simulink model "PrkgClimaEveMgr"  (R2022b) (continued)
== system PrkgClimaEveMgr == (continued)
  ClimaCfgForPostCdnAvl1_ClimaCfgForPostCdnAvl1/1 -> PrkgClimaEveMgr_Runnable_sys/15(ClimaCfgForPostCdnAvl1_ClimaCfgForPostCdnAvl1_read)
  ClimaCfgForPreClng1_ClimaCfgForPreClng1/1 -> PrkgClimaEveMgr_Runnable_sys/16(ClimaCfgForPreClng1_ClimaCfgForPreClng1_read)
  ClimaCfgForVehElec1_ClimaCfgForVehElec1/1 -> PrkgClimaEveMgr_Runnable_sys/17(ClimaCfgForVehElec1_ClimaCfgForVehElec1_read)
  ClimaCfgForVehHyb1_ClimaCfgForVehHyb1/1 -> PrkgClimaEveMgr_Runnable_sys/18(ClimaCfgForVehHyb1_ClimaCfgForVehHyb1_read)
  ClimaCfgForVrntOfVehSysElec1_ClimaCfgForVrntOfVehSysElec1/1 -> PrkgClimaEveMgr_Runnable_sys/19(ClimaCfgForVrntOfVehSysElec1_ClimaCfgForVrntOfVehSysElec1_read)
  ClimaOvrHeatProWarn_ClimaOvrHeatProWarn/1 -> PrkgClimaEveMgr_Runnable_sys/20(ClimaOvrHeatProWarn_ClimaOvrHeatProWarn_read)
  ClimaOvrHeatReqFromExrsc_ClimaOvrHeatReqFromExrsc/1 -> PrkgClimaEveMgr_Runnable_sys/21(ClimaOvrHeatReqFromExrsc_ClimaOvrHeatReqFromExrsc_read)
  ClimaStrtTiDly_ClimaStrtTiDly/1 -> PrkgClimaEveMgr_Runnable_sys/22(ClimaStrtTiDly_ClimaStrtTiDly_read)
  ClimaTmrSts_ClimaTmrSts/1 -> PrkgClimaEveMgr_Runnable_sys/23(ClimaTmrSts_ClimaTmrSts_read)
  CmptmtClsd_CmptmtClsd/1 -> PrkgClimaEveMgr_Runnable_sys/24(CmptmtClsd_CmptmtClsd_read)
  COHSts_COHSts/1 -> PrkgClimaEveMgr_Runnable_sys/25(COHSts_COHSts_read)
  CooltTForPrkgClima_CooltTForPrkgClima/1 -> PrkgClimaEveMgr_Runnable_sys/26(CooltTForPrkgClima_CooltTForPrkgClima_read)
  DCChrgnHndlSts_DCChrgnHndlSts/1 -> PrkgClimaEveMgr_Runnable_sys/27(DCChrgnHndlSts_DCChrgnHndlSts_read)
  DlyReqForTmrForPrkgClima_DlyReqForTmrForPrkgClima/1 -> PrkgClimaEveMgr_Runnable_sys/28(DlyReqForTmrForPrkgClima_DlyReqForTmrForPrkgClima_read)
  DshbTForPrkgClima_DshbTForPrkgClima/1 -> PrkgClimaEveMgr_Runnable_sys/29(DshbTForPrkgClima_DshbTForPrkgClima_read)
  EngT_Ngcc_EngT/1 -> PrkgClimaEveMgr_Runnable_sys/30(EngT_Ngcc_EngT_read)
  ErsCmdByKeyFromExsrc_ErsCmdByKeyFromExsrc/1 -> PrkgClimaEveMgr_Runnable_sys/31(ErsCmdByKeyFromExsrc_ErsCmdByKeyFromExsrc_read)
  ErsRunTimeFromExtSrc_ErsRunTimeFromExtSrc/1 -> PrkgClimaEveMgr_Runnable_sys/32(ErsRunTimeFromExtSrc_ErsRunTimeFromExtSrc_read)
  HeatrElecNotAvlForTmr_HeatrElecNotAvlForTmr/1 -> PrkgClimaEveMgr_Runnable_sys/33(HeatrElecNotAvlForTmr_HeatrElecNotAvlForTmr_read)
  HeatrTypSetgForPrkgClima_HeatrTypSetgForPrkgClima/1 -> PrkgClimaEveMgr_Runnable_sys/34(HeatrTypSetgForPrkgClima_HeatrTypSetgForPrkgClima_read)
  HeatUpRqrdForPrkgClima_HeatUpRqrdForPrkgClima/1 -> PrkgClimaEveMgr_Runnable_sys/35(HeatUpRqrdForPrkgClima_HeatUpRqrdForPrkgClima_read)
  HMIExtVentFanVolReqFromExtSrc_HMIExtVentFanVolReqFromExtSrc/1 -> PrkgClimaEveMgr_Runnable_sys/36(HMIExtVentFanVolReqFromExtSrc_HMIExtVentFanVolReqFromExtSrc_read)
  HMIExtVentonoffReqFromExtSrc_HMIExtVentonoffReqFromExtSrc/1 -> PrkgClimaEveMgr_Runnable_sys/37(HMIExtVentonoffReqFromExtSrc_HMIExtVentonoffReqFromExtSrc_read)
  HMIExtVentTSpReqFromExtSrc_HMIExtVentTSpReqFromExtSrc/1 -> PrkgClimaEveMgr_Runnable_sys/38(HMIExtVentTSpReqFromExtSrc_HMIExtVentTSpReqFromExtSrc_read)
  HMIExtVentTStsReqFromExtSrc_HMIExtVentTStsReqFromExtSrc/1 -> PrkgClimaEveMgr_Runnable_sys/39(HMIExtVentTStsReqFromExtSrc_HMIExtVentTStsReqFromExtSrc_read)
  HvBattSoc_HvBattSoc/1 -> PrkgClimaEveMgr_Runnable_sys/40(HvBattSoc_HvBattSoc_read)
  HvSysCnctWoU_HvSysCnctWoU/1 -> PrkgClimaEveMgr_Runnable_sys/41(HvSysCnctWoU_HvSysCnctWoU_read)
  HvSysWoU_HvSysWoU/1 -> PrkgClimaEveMgr_Runnable_sys/42(HvSysWoU_HvSysWoU_read)
  IntCo2ActvSts_IntCo2ActvSts/1 -> PrkgClimaEveMgr_Runnable_sys/43(IntCo2ActvSts_IntCo2ActvSts_read)
  IntCo2fromExsrc_IntCo2fromExsrc/1 -> PrkgClimaEveMgr_Runnable_sys/44(IntCo2fromExsrc_IntCo2fromExsrc_read)
  KeyClimaActv_KeyClimaActv/1 -> PrkgClimaEveMgr_Runnable_sys/45(KeyClimaActv_KeyClimaActv_read)
  LockgCenSts_Ngcc_LockgCenSts/1 -> PrkgClimaEveMgr_Runnable_sys/46(LockgCenSts_Ngcc_LockgCenSts_read)
  OnBdChrgrHndlSts_OnBdChrgrHndlSts/1 -> PrkgClimaEveMgr_Runnable_sys/47(OnBdChrgrHndlSts_OnBdChrgrHndlSts_read)
  PostDrvgClimaReq_Ngcc_PostDrvgClimaReq/1 -> PrkgClimaEveMgr_Runnable_sys/48(PostDrvgClimaReq_Ngcc_PostDrvgClimaReq_read)
  PreClngRqrdFromExtSrc_PreClngRqrdFromExtSrc/1 -> PrkgClimaEveMgr_Runnable_sys/49(PreClngRqrdFromExtSrc_PreClngRqrdFromExtSrc_read)
  PrkgClimaDiByCarMod_PrkgClimaDiByCarMod/1 -> PrkgClimaEveMgr_Runnable_sys/50(PrkgClimaDiByCarMod_PrkgClimaDiByCarMod_read)
  PrkgClimaRqrdImdtForBattULo_PrkgClimaRqrdImdtForBattULo/1 -> PrkgClimaEveMgr_Runnable_sys/51(PrkgClimaRqrdImdtForBattULo_PrkgClimaRqrdImdtForBattULo_read)
  PrkgClimaRqrdImdtFromActvMgr_PrkgClimaRqrdImdtFromActvMgr/1 -> PrkgClimaEveMgr_Runnable_sys/52(PrkgClimaRqrdImdtFromActvMgr_PrkgClimaRqrdImdtFromActvMgr_read)
  PrkgClimaRqrdImdtFromExtSrc_PrkgClimaRqrdImdtFromExtSrc/1 -> PrkgClimaEveMgr_Runnable_sys/53(PrkgClimaRqrdImdtFromExtSrc_PrkgClimaRqrdImdtFromExtSrc_read)
  PrkgClimaRqrdTmrForBattULo_PrkgClimaRqrdTmrForBattULo/1 -> PrkgClimaEveMgr_Runnable_sys/54(PrkgClimaRqrdTmrForBattULo_PrkgClimaRqrdTmrForBattULo_read)
  RemClimaFnshd_RemClimaFnshd/1 -> PrkgClimaEveMgr_Runnable_sys/55(RemClimaFnshd_RemClimaFnshd_read)
  RemClimaReqFromExtSrc_RemClimaReqFromExtSrc/1 -> PrkgClimaEveMgr_Runnable_sys/56(RemClimaReqFromExtSrc_RemClimaReqFromExtSrc_read)
  RemStrtExtnTiReqFromExtSrc_RemStrtExtnTiReqFromExtSrc/1 -> PrkgClimaEveMgr_Runnable_sys/57(RemStrtExtnTiReqFromExtSrc_RemStrtExtnTiReqFromExtSrc_read)
  RemStrtHvCtrlReqFromExtSrc_RemStrtHvCtrlReqFromExtSrc/1 -> PrkgClimaEveMgr_Runnable_sys/58(RemStrtHvCtrlReqFromExtSrc_RemStrtHvCtrlReqFromExtSrc_read)
  RemVentFbSts_RemVentFbSts/1 -> PrkgClimaEveMgr_Runnable_sys/59(RemVentFbSts_RemVentFbSts_read)
  RemVentReqfromExsrc_RemVentReqfromExsrc/1 -> PrkgClimaEveMgr_Runnable_sys/60(RemVentReqfromExsrc_RemVentReqfromExsrc_read)
  ReqForPrkgClima_ReqForPrkgClima/1 -> PrkgClimaEveMgr_Runnable_sys/61(ReqForPrkgClima_ReqForPrkgClima_read)
  ReqForSwtHeatrTypFromActvMgr_ReqForSwtHeatrTypFromActvMgr/1 -> PrkgClimaEveMgr_Runnable_sys/62(ReqForSwtHeatrTypFromActvMgr_ReqForSwtHeatrTypFromActvMgr_read)
  RtSdlForPrkgClima_RtSdlForPrkgClima/1 -> PrkgClimaEveMgr_Runnable_sys/63(RtSdlForPrkgClima_RtSdlForPrkgClima_read)
  SeatHeatgEnadFromHmi_SeatHeatgEnadFromHmi/1 -> PrkgClimaEveMgr_Runnable_sys/64(SeatHeatgEnadFromHmi_SeatHeatgEnadFromHmi_read)
  SeatHeatgEnadFromTelm_SeatHeatgEnadFromTelm/1 -> PrkgClimaEveMgr_Runnable_sys/65(SeatHeatgEnadFromTelm_SeatHeatgEnadFromTelm_read)
  SnsrQfOkForPrkgClima_SnsrQfOkForPrkgClima/1 -> PrkgClimaEveMgr_Runnable_sys/66(SnsrQfOkForPrkgClima_SnsrQfOkForPrkgClima_read)
  SteerWhlHeatgDurgClimaEnadFromExsrc_SteerWhlHeatgDurgClimaEnadFromExsrc/1 -> PrkgClimaEveMgr_Runnable_sys/67(SteerWhlHeatgDurgClimaEnadFromExsrc_SteerWhlHeatgDurgClimaEnadFromExsrc_read)
  SteerWhlHeatgEnadFromHmi_SteerWhlHeatgEnadFromHmi/1 -> PrkgClimaEveMgr_Runnable_sys/68(SteerWhlHeatgEnadFromHmi_SteerWhlHeatgEnadFromHmi_read)
  SteerWhlHeatgEnadFromTelm_SteerWhlHeatgEnadFromTelm/1 -> PrkgClimaEveMgr_Runnable_sys/69(SteerWhlHeatgEnadFromTelm_SteerWhlHeatgEnadFromTelm_read)
  StrtRemStsFromExsrc_StrtRemStsFromExsrc/1 -> PrkgClimaEveMgr_Runnable_sys/70(StrtRemStsFromExsrc_StrtRemStsFromExsrc_read)
  SuspStrtActvForPrkgClima_SuspStrtActvForPrkgClima/1 -> PrkgClimaEveMgr_Runnable_sys/71(SuspStrtActvForPrkgClima_SuspStrtActvForPrkgClima_read)
  SysDogSlpCountTi_SysDogSlpCountTi/1 -> PrkgClimaEveMgr_Runnable_sys/72(SysDogSlpCountTi_SysDogSlpCountTi_read)
  TelmClimaExtVentnLvlFromExtSrc_TelmClimaExtVentnLvlFromExtSrc/1 -> PrkgClimaEveMgr_Runnable_sys/73(TelmClimaExtVentnLvlFromExtSrc_TelmClimaExtVentnLvlFromExtSrc_read)
  TelmClimaExtVentnReqFromExtSrc_TelmClimaExtVentnReqFromExtSrc/1 -> PrkgClimaEveMgr_Runnable_sys/74(TelmClimaExtVentnReqFromExtSrc_TelmClimaExtVentnReqFromExtSrc_read)
  TelmClimaExtVentnTempRangeFromExtSrc_TelmClimaExtVentnTempRangeFromExtSrc/1 -> PrkgClimaEveMgr_Runnable_sys/75(TelmClimaExtVentnTempRangeFromExtSrc_TelmClimaExtVentnTempRangeFromExtSrc_read)
  TelmClimaExtVentnTSpSpclFromExtSrc_TelmClimaExtVentnTSpSpclFromExtSrc/1 -> PrkgClimaEveMgr_Runnable_sys/76(TelmClimaExtVentnTSpSpclFromExtSrc_TelmClimaExtVentnTSpSpclFromExtSrc_read)
  TelmClimaTmrFromExtSrc_TelmClimaTmrFromExtSrc/1 -> PrkgClimaEveMgr_Runnable_sys/77(TelmClimaTmrFromExtSrc_TelmClimaTmrFromExtSrc_read)
  TelmSeatDrvrHeatReqFromExsrc_TelmSeatDrvrHeatReqFromExsrc/1 -> PrkgClimaEveMgr_Runnable_sys/78(TelmSeatDrvrHeatReqFromExsrc_TelmSeatDrvrHeatReqFromExsrc_read)
  TelmSeatDrvVentnReqFromExsrc_TelmSeatDrvVentnReqFromExsrc/1 -> PrkgClimaEveMgr_Runnable_sys/79(TelmSeatDrvVentnReqFromExsrc_TelmSeatDrvVentnReqFromExsrc_read)
  TelmSeatPassHeatReqFromExsrc_TelmSeatPassHeatReqFromExsrc/1 -> PrkgClimaEveMgr_Runnable_sys/80(TelmSeatPassHeatReqFromExsrc_TelmSeatPassHeatReqFromExsrc_read)
  TelmSeatPassVentnReqFromExsrc_TelmSeatPassVentnReqFromExsrc/1 -> PrkgClimaEveMgr_Runnable_sys/81(TelmSeatPassVentnReqFromExsrc_TelmSeatPassVentnReqFromExsrc_read)
  TelmSeatSecLeHeatReqFromExsrc_TelmSeatSecLeHeatReqFromExsrc/1 -> PrkgClimaEveMgr_Runnable_sys/82(TelmSeatSecLeHeatReqFromExsrc_TelmSeatSecLeHeatReqFromExsrc_read)
  TelmSeatSecLeVentnReqFromExsrc_TelmSeatSecLeVentnReqFromExsrc/1 -> PrkgClimaEveMgr_Runnable_sys/83(TelmSeatSecLeVentnReqFromExsrc_TelmSeatSecLeVentnReqFromExsrc_read)
  TelmSeatSecRiHeatReqFromExsrc_TelmSeatSecRiHeatReqFromExsrc/1 -> PrkgClimaEveMgr_Runnable_sys/84(TelmSeatSecRiHeatReqFromExsrc_TelmSeatSecRiHeatReqFromExsrc_read)
  TelmSeatSecRiVentnReqFromExsrc_TelmSeatSecRiVentnReqFromExsrc/1 -> PrkgClimaEveMgr_Runnable_sys/85(TelmSeatSecRiVentnReqFromExsrc_TelmSeatSecRiVentnReqFromExsrc_read)
  TForHeatrPreCdngTyp_TForHeatrPreCdngTyp/1 -> PrkgClimaEveMgr_Runnable_sys/86(TForHeatrPreCdngTyp_TForHeatrPreCdngTyp_read)
  TmrForPrkgClima_TmrForPrkgClima/1 -> PrkgClimaEveMgr_Runnable_sys/87(TmrForPrkgClima_TmrForPrkgClima_read)
  VehModMngtGlbSafe1_Ngcc_VehModMngtGlbSafe1/1 -> PrkgClimaEveMgr_Runnable_sys/88(VehModMngtGlbSafe1_Ngcc_VehModMngtGlbSafe1_read)
  VehSunLoadTot_VehSunLoadTot/1 -> PrkgClimaEveMgr_Runnable_sys/89(VehSunLoadTot_VehSunLoadTot_read)
  VehSunLoadTotFild_VehSunLoadTotFild/1 -> PrkgClimaEveMgr_Runnable_sys/90(VehSunLoadTotFild_VehSunLoadTotFild_read)
  ClimaCfgForStatrtDevice_ClimaCfgForStartDevice/1 -> PrkgClimaEveMgr_Runnable_sys/91(ClimaCfgForStatrtDevice_ClimaCfgForStartDevice_read)
  PostHeatgRunTiEstimd_PostHeatgRunTiEstimd/1 -> PrkgClimaEveMgr_Runnable_sys/92(PostHeatgRunTiEstimd_PostHeatgRunTiEstimd_read)
  TelmDefrostReqFromExsrc_TelmDefrostReqFromExsrc/1 -> PrkgClimaEveMgr_Runnable_sys/93(TelmDefrostReqFromExsrc_TelmDefrostReqFromExsrc_read)
  TelmSeatThrdLeHeatReqFromExsrc_TelmSeatThrdLeHeatReqFromExsrc/1 -> PrkgClimaEveMgr_Runnable_sys/94(TelmSeatThrdLeHeatReqFromExsrc_TelmSeatThrdLeHeatReqFromExsrc_read)
  TelmSeatThrdLeVentnReqFromExsrc_TelmSeatThrdLeVentnReqFromExsrc/1 -> PrkgClimaEveMgr_Runnable_sys/95(TelmSeatThrdLeVentnReqFromExsrc_TelmSeatThrdLeVentnReqFromExsrc_read)
  TelmSeatThrdRiHeatReqFromExsrc_TelmSeatThrdRiHeatReqFromExsrc/1 -> PrkgClimaEveMgr_Runnable_sys/96(TelmSeatThrdRiHeatReqFromExsrc_TelmSeatThrdRiHeatReqFromExsrc_read)
  TelmSeatThrdRiVentnReqFromExsrc_TelmSeatThrdRiVentnReqFromExsrc/1 -> PrkgClimaEveMgr_Runnable_sys/97(TelmSeatThrdRiVentnReqFromExsrc_TelmSeatThrdRiVentnReqFromExsrc_read)
  TempRangeFromExtSrc_TempRangeFromExtSrc/1 -> PrkgClimaEveMgr_Runnable_sys/98(TempRangeFromExtSrc_TempRangeFromExtSrc_read)
  CmptmtTFrntRaw_CmptmtTFrntRaw/1 -> PrkgClimaEveMgr_Runnable_sys/99(CmptmtTFrntRaw_CmptmtTFrntRaw_read)
  BookTrvlClimaActvReq2FromExSrc_BookTrvlClimaActvReq2FromExSrc/1 -> PrkgClimaEveMgr_Runnable_sys/100(BookTrvlClimaActvReq2FromExSrc_BookTrvlClimaActvReq2FromExSrc_read)
  BookTrvlClimaActvReq3FromExSrc_BookTrvlClimaActvReq3FromExSrc/1 -> PrkgClimaEveMgr_Runnable_sys/101(BookTrvlClimaActvReq3FromExSrc_BookTrvlClimaActvReq3FromExSrc_read)
  BookTrvlClimaActvReq4FromExSrc_BookTrvlClimaActvReq4FromExSrc/1 -> PrkgClimaEveMgr_Runnable_sys/102(BookTrvlClimaActvReq4FromExSrc_BookTrvlClimaActvReq4FromExSrc_read)
  BookTrvlClimaActvReq5FromExSrc_BookTrvlClimaActvReq5FromExSrc/1 -> PrkgClimaEveMgr_Runnable_sys/103(BookTrvlClimaActvReq5FromExSrc_BookTrvlClimaActvReq5FromExSrc_read)
  BookTrvlClimaActvReq6FromExSrc_BookTrvlClimaActvReq6FromExSrc/1 -> PrkgClimaEveMgr_Runnable_sys/104(BookTrvlClimaActvReq6FromExSrc_BookTrvlClimaActvReq6FromExSrc_read)
  BookTrvlClimaActvReq7FromExSrc_BookTrvlClimaActvReq7FromExSrc/1 -> PrkgClimaEveMgr_Runnable_sys/105(BookTrvlClimaActvReq7FromExSrc_BookTrvlClimaActvReq7FromExSrc_read)
  BookTrvlClimaFnshd_BookTrvlClimaFnshd/1 -> PrkgClimaEveMgr_Runnable_sys/106(BookTrvlClimaFnshd_BookTrvlClimaFnshd_read)
  ClimaActv_Ngcc_ClimaActv/1 -> PrkgClimaEveMgr_Runnable_sys/107(ClimaActv_Ngcc_ClimaActv_read)
  ClimaCfgForAirMtrlSnsrPrsnt1_ClimaCfgForAirMtrlSnsrPrsnt1/1 -> PrkgClimaEveMgr_Runnable_sys/108(ClimaCfgForAirMtrlSnsrPrsnt1_ClimaCfgForAirMtrlSnsrPrsnt1_read)
  ClimaCfgForBRAND_ClimaCfgForBRAND/1 -> PrkgClimaEveMgr_Runnable_sys/109(ClimaCfgForBRAND_ClimaCfgForBRAND_read)
  ClimaCfgForCabinOxgenSupply_ClimaCfgForCabinOxgenSupply/1 -> PrkgClimaEveMgr_Runnable_sys/110(ClimaCfgForCabinOxgenSupply_ClimaCfgForCabinOxgenSupply_read)
  ClimaCfgForHvacTyp_ClimaCfgForHvacTyp/1 -> PrkgClimaEveMgr_Runnable_sys/111(ClimaCfgForHvacTyp_ClimaCfgForHvacTyp_read)
  ClimaCfgForLifeDetection_ClimaCfgForLifeDetection/1 -> PrkgClimaEveMgr_Runnable_sys/112(ClimaCfgForLifeDetection_ClimaCfgForLifeDetection_read)
  ClimaCfgForPrpsnTyp_ClimaCfgForPrpsnTyp/1 -> PrkgClimaEveMgr_Runnable_sys/113(ClimaCfgForPrpsnTyp_ClimaCfgForPrpsnTyp_read)
  ClimaCfgForRFKEY_ClimaCfgForRFKEY/1 -> PrkgClimaEveMgr_Runnable_sys/114(ClimaCfgForRFKEY_ClimaCfgForRFKEY_read)
  ClimaCfgForSnsrHumPrsnt1_ClimaCfgForSnsrHumPrsnt1/1 -> PrkgClimaEveMgr_Runnable_sys/115(ClimaCfgForSnsrHumPrsnt1_ClimaCfgForSnsrHumPrsnt1_read)
  ClimaCfgForVehTyp_ClimaCfgForVehTyp/1 -> PrkgClimaEveMgr_Runnable_sys/116(ClimaCfgForVehTyp_ClimaCfgForVehTyp_read)
  ClimaWakUpTiDif1FromExSrc_ClimaWakUpTiDif1FromExSrc/1 -> PrkgClimaEveMgr_Runnable_sys/117(ClimaWakUpTiDif1FromExSrc_ClimaWakUpTiDif1FromExSrc_read)
  ClimaWakUpTiDif2FromExSrc_ClimaWakUpTiDif2FromExSrc/1 -> PrkgClimaEveMgr_Runnable_sys/118(ClimaWakUpTiDif2FromExSrc_ClimaWakUpTiDif2FromExSrc_read)
  ClimaWakUpTiDif3FromExSrc_ClimaWakUpTiDif3FromExSrc/1 -> PrkgClimaEveMgr_Runnable_sys/119(ClimaWakUpTiDif3FromExSrc_ClimaWakUpTiDif3FromExSrc_read)
  ClimaWakUpTiDif4FromExSrc_ClimaWakUpTiDif4FromExSrc/1 -> PrkgClimaEveMgr_Runnable_sys/120(ClimaWakUpTiDif4FromExSrc_ClimaWakUpTiDif4FromExSrc_read)
  ClimaWakUpTiDif5FromExSrc_ClimaWakUpTiDif5FromExSrc/1 -> PrkgClimaEveMgr_Runnable_sys/121(ClimaWakUpTiDif5FromExSrc_ClimaWakUpTiDif5FromExSrc_read)
  ClimaWakUpTiDif6FromExSrc_ClimaWakUpTiDif6FromExSrc/1 -> PrkgClimaEveMgr_Runnable_sys/122(ClimaWakUpTiDif6FromExSrc_ClimaWakUpTiDif6FromExSrc_read)
  ClimaWakUpTiDif7FromExSrc_ClimaWakUpTiDif7FromExSrc/1 -> PrkgClimaEveMgr_Runnable_sys/123(ClimaWakUpTiDif7FromExSrc_ClimaWakUpTiDif7FromExSrc_read)
  CmptmtAirTEstimdAtRowFirst_CmptmtAirTEstimdAtRowFirst/1 -> PrkgClimaEveMgr_Runnable_sys/124(CmptmtAirTEstimdAtRowFirst_CmptmtAirTEstimdAtRowFirst_read)
  CmptmtClngActv_CmptmtClngActv/1 -> PrkgClimaEveMgr_Runnable_sys/125(CmptmtClngActv_CmptmtClngActv_read)
  CmptmtRelHum_Ngcc_CmptmtRelHum/1 -> PrkgClimaEveMgr_Runnable_sys/126(CmptmtRelHum_Ngcc_CmptmtRelHum_read)
  CmptmtTRearRaw_CmptmtTRearRaw/1 -> PrkgClimaEveMgr_Runnable_sys/127(CmptmtTRearRaw_CmptmtTRearRaw_read)
  DoorOpenStsForClima_DoorOpenStsForClima/1 -> PrkgClimaEveMgr_Runnable_sys/128(DoorOpenStsForClima_DoorOpenStsForClima_read)
  DoorStsWithOutTrForClima_DoorStsWithOutTrForClima/1 -> PrkgClimaEveMgr_Runnable_sys/129(DoorStsWithOutTrForClima_DoorStsWithOutTrForClima_read)
  EngSt1WdStsFromExsrc_EngSt1WdStsFromExsrc/1 -> PrkgClimaEveMgr_Runnable_sys/130(EngSt1WdStsFromExsrc_EngSt1WdStsFromExsrc_read)
  FrntHvacBlowerSts_FrntHvacBlowerSts/1 -> PrkgClimaEveMgr_Runnable_sys/131(FrntHvacBlowerSts_FrntHvacBlowerSts_read)
  GearLvrIndcn_GearLvrIndcn/1 -> PrkgClimaEveMgr_Runnable_sys/132(GearLvrIndcn_GearLvrIndcn_read)
  GearLvrIndcn_GearLvrIndcn_IsUpdated/1 -> PrkgClimaEveMgr_Runnable_sys/133(GearLvrIndcn_GearLvrIndcn_IsUpdated_read)
  HmiWinterLockCarPrevnIceSetNgcc_HmiWinterLockCarPrevnIceSetNgcc/1 -> PrkgClimaEveMgr_Runnable_sys/134(HmiWinterLockCarPrevnIceSetNgcc_HmiWinterLockCarPrevnIceSetNgcc_read)
  HvBattMntnReq_HvBattMntnReq/1 -> PrkgClimaEveMgr_Runnable_sys/135(HvBattMntnReq_HvBattMntnReq_read)
  HvBattMntnReq_HvBattMntnReq_IsUpdated/1 -> PrkgClimaEveMgr_Runnable_sys/136(HvBattMntnReq_HvBattMntnReq_IsUpdated_read)
  InflateAirSrcSwtNgcc_InflateAirSrcSwtNgcc/1 -> PrkgClimaEveMgr_Runnable_sys/137(InflateAirSrcSwtNgcc_InflateAirSrcSwtNgcc_read)
  KeyClimaActvFnshd_KeyClimaActvFnshd/1 -> PrkgClimaEveMgr_Runnable_sys/138(KeyClimaActvFnshd_KeyClimaActvFnshd_read)
  LonglifeBatteryReqNgcc_LonglifeBatteryReqNgcc/1 -> PrkgClimaEveMgr_Runnable_sys/139(LonglifeBatteryReqNgcc_LonglifeBatteryReqNgcc_read)
  MemReadForPrkgClimaEveMgr_MemReadForPrkgClimaEveMgr/1 -> PrkgClimaEveMgr_Runnable_sys/140(MemReadForPrkgClimaEveMgr_MemReadForPrkgClimaEveMgr_read)
  OGMAirSrcFnshd_OGMAirSrcFnshd/1 -> PrkgClimaEveMgr_Runnable_sys/141(OGMAirSrcFnshd_OGMAirSrcFnshd_read)
  PrvntWinFrz_PrvntWinFrz/1 -> PrkgClimaEveMgr_Runnable_sys/142(PrvntWinFrz_PrvntWinFrz_read)
  PrvntWinFrzFnshd_PrvntWinFrzFnshd/1 -> PrkgClimaEveMgr_Runnable_sys/143(PrvntWinFrzFnshd_PrvntWinFrzFnshd_read)
  RemClimaFnshd1_RemClimaFnshd1/1 -> PrkgClimaEveMgr_Runnable_sys/144(RemClimaFnshd1_RemClimaFnshd1_read)
  RemClimaWarn_RemClimaWarn/1 -> PrkgClimaEveMgr_Runnable_sys/145(RemClimaWarn_RemClimaWarn_read)
  RemOxygenSplyFnshd_RemOxygenSplyFnshd/1 -> PrkgClimaEveMgr_Runnable_sys/146(RemOxygenSplyFnshd_RemOxygenSplyFnshd_read)
  RemOxygenSplyFnshd1_RemOxygenSplyFnshd1/1 -> PrkgClimaEveMgr_Runnable_sys/147(RemOxygenSplyFnshd1_RemOxygenSplyFnshd1_read)
  RemOxygenSplyWarn_RemOxygenSplyWarn/1 -> PrkgClimaEveMgr_Runnable_sys/148(RemOxygenSplyWarn_RemOxygenSplyWarn_read)
  TcsOvrheatdProtnStsNgcc_TcsOvrheatdProtnStsNgcc/1 -> PrkgClimaEveMgr_Runnable_sys/149(TcsOvrheatdProtnStsNgcc_TcsOvrheatdProtnStsNgcc_read)
  TelmBookClimaReqHmiCmptmtTSpSpclFromExtSrc_TelmBookClimaReqHmiCmptmtTSpSpclFromExtSrc/1 -> PrkgClimaEveMgr_Runnable_sys/150(TelmBookClimaReqHmiCmptmtTSpSpclFromExtSrc_TelmBookClimaReqHmiCmptmtTSpSpclFromExtSrc_read)
  TelmBookClimaReqOnOffNoReqFromExtSrc_TelmBookClimaReqOnOffNoReqFromExtSrc/1 -> PrkgClimaEveMgr_Runnable_sys/151(TelmBookClimaReqOnOffNoReqFromExtSrc_TelmBookClimaReqOnOffNoReqFromExtSrc_read)
  TelmBookClimaReqTempRangeFromExtSrc_TelmBookClimaReqTempRangeFromExtSrc/1 -> PrkgClimaEveMgr_Runnable_sys/152(TelmBookClimaReqTempRangeFromExtSrc_TelmBookClimaReqTempRangeFromExtSrc_read)
  TelmBookClimaTmrFromExtSrc_TelmBookClimaTmrFromExtSrc/1 -> PrkgClimaEveMgr_Runnable_sys/153(TelmBookClimaTmrFromExtSrc_TelmBookClimaTmrFromExtSrc_read)
  TelmBookSeatHeatClimalReqDrvHeatClimaLvlFromExtSrc_TelmBookSeatHeatClimalReqDrvHeatClimaLvlFromExtSrc/1 -> PrkgClimaEveMgr_Runnable_sys/154(TelmBookSeatHeatClimalReqDrvHeatClimaLvlFromExtSrc_TelmBookSeatHeatClimalReqDrvHeatClimaLvlFromExtSrc_read)
  TelmBookSeatHeatClimalReqPassHeatClimaLvlFromExtSrc_TelmBookSeatHeatClimalReqPassHeatClimaLvlFromExtSrc/1 -> PrkgClimaEveMgr_Runnable_sys/155(TelmBookSeatHeatClimalReqPassHeatClimaLvlFromExtSrc_TelmBookSeatHeatClimalReqPassHeatClimaLvlFromExtSrc_read)
  TelmBookSeatHeatClimalReqSecLeHeatClimaLvlFromExtSrc_TelmBookSeatHeatClimalReqSecLeHeatClimaLvlFromExtSrc/1 -> PrkgClimaEveMgr_Runnable_sys/156(TelmBookSeatHeatClimalReqSecLeHeatClimaLvlFromExtSrc_TelmBookSeatHeatClimalReqSecLeHeatClimaLvlFromExtSrc_read)
  TelmBookSeatHeatClimalReqSecRiHeatClimaLvlFromExtSrc_TelmBookSeatHeatClimalReqSecRiHeatClimaLvlFromExtSrc/1 -> PrkgClimaEveMgr_Runnable_sys/157(TelmBookSeatHeatClimalReqSecRiHeatClimaLvlFromExtSrc_TelmBookSeatHeatClimalReqSecRiHeatClimaLvlFromExtSrc_read)
  TelmBookSeatHeatClimalReqThrdLeHeatClimaLvlFromExtSrc_TelmBookSeatHeatClimalReqThrdLeHeatClimaLvlFromExtSrc/1 -> PrkgClimaEveMgr_Runnable_sys/158(TelmBookSeatHeatClimalReqThrdLeHeatClimaLvlFromExtSrc_TelmBookSeatHeatClimalReqThrdLeHeatClimaLvlFromExtSrc_read)
  TelmBookSeatHeatClimalReqThrdRiHeatClimaLvlFromExtSrc_TelmBookSeatHeatClimalReqThrdRiHeatClimaLvlFromExtSrc/1 -> PrkgClimaEveMgr_Runnable_sys/159(TelmBookSeatHeatClimalReqThrdRiHeatClimaLvlFromExtSrc_TelmBookSeatHeatClimalReqThrdRiHeatClimaLvlFromExtSrc_read)
  TelmBookSeatVentnClimalReqDrvVentnClimaLvlFromExtSrc_TelmBookSeatVentnClimalReqDrvVentnClimaLvlFromExtSrc/1 -> PrkgClimaEveMgr_Runnable_sys/160(TelmBookSeatVentnClimalReqDrvVentnClimaLvlFromExtSrc_TelmBookSeatVentnClimalReqDrvVentnClimaLvlFromExtSrc_read)
  TelmBookSeatVentnClimalReqPassVentnClimaLvlFromExtSrc_TelmBookSeatVentnClimalReqPassVentnClimaLvlFromExtSrc/1 -> PrkgClimaEveMgr_Runnable_sys/161(TelmBookSeatVentnClimalReqPassVentnClimaLvlFromExtSrc_TelmBookSeatVentnClimalReqPassVentnClimaLvlFromExtSrc_read)
  TelmBookSeatVentnClimalReqSecLeVentnClimaLvlFromExtSrc_TelmBookSeatVentnClimalReqSecLeVentnClimaLvlFromExtSrc/1 -> PrkgClimaEveMgr_Runnable_sys/162(TelmBookSeatVentnClimalReqSecLeVentnClimaLvlFromExtSrc_TelmBookSeatVentnClimalReqSecLeVentnClimaLvlFromExtSrc_read)
  TelmBookSeatVentnClimalReqSecRiVentnClimaLvlFromExtSrc_TelmBookSeatVentnClimalReqSecRiVentnClimaLvlFromExtSrc/1 -> PrkgClimaEveMgr_Runnable_sys/163(TelmBookSeatVentnClimalReqSecRiVentnClimaLvlFromExtSrc_TelmBookSeatVentnClimalReqSecRiVentnClimaLvlFromExtSrc_read)
  TelmBookSeatVentnClimalReqThrdLeVentnClimaLvlFromExtSrc_TelmBookSeatVentnClimalReqThrdLeVentnClimaLvlFromExtSrc/1 -> PrkgClimaEveMgr_Runnable_sys/164(TelmBookSeatVentnClimalReqThrdLeVentnClimaLvlFromExtSrc_TelmBookSeatVentnClimalReqThrdLeVentnClimaLvlFromExtSrc_read)
  TelmBookSeatVentnClimalReqThrdRiVentnClimaLvlFromExtSrc_TelmBookSeatVentnClimalReqThrdRiVentnClimaLvlFromExtSrc/1 -> PrkgClimaEveMgr_Runnable_sys/165(TelmBookSeatVentnClimalReqThrdRiVentnClimaLvlFromExtSrc_TelmBookSeatVentnClimalReqThrdRiVentnClimaLvlFromExtSrc_read)
  TelmBookSteerWhlHeatClimalReqFromExtSrc_TelmBookSteerWhlHeatClimalReqFromExtSrc/1 -> PrkgClimaEveMgr_Runnable_sys/166(TelmBookSteerWhlHeatClimalReqFromExtSrc_TelmBookSteerWhlHeatClimalReqFromExtSrc_read)
  TelmDefrostFnshd_TelmDefrostFnshd/1 -> PrkgClimaEveMgr_Runnable_sys/167(TelmDefrostFnshd_TelmDefrostFnshd_read)
  TelmOxygenReqFromExSrc_TelmOxygenReqFromExSrc/1 -> PrkgClimaEveMgr_Runnable_sys/168(TelmOxygenReqFromExSrc_TelmOxygenReqFromExSrc_read)
  TelmSeatHeatDrvrFnshd_TelmSeatHeatDrvrFnshd/1 -> PrkgClimaEveMgr_Runnable_sys/169(TelmSeatHeatDrvrFnshd_TelmSeatHeatDrvrFnshd_read)
  TelmSeatHeatPassFnshd_TelmSeatHeatPassFnshd/1 -> PrkgClimaEveMgr_Runnable_sys/170(TelmSeatHeatPassFnshd_TelmSeatHeatPassFnshd_read)
  TelmSeatHeatSecLeFnshd_TelmSeatHeatSecLeFnshd/1 -> PrkgClimaEveMgr_Runnable_sys/171(TelmSeatHeatSecLeFnshd_TelmSeatHeatSecLeFnshd_read)
  TelmSeatHeatSecRiFnshd_TelmSeatHeatSecRiFnshd/1 -> PrkgClimaEveMgr_Runnable_sys/172(TelmSeatHeatSecRiFnshd_TelmSeatHeatSecRiFnshd_read)
  TelmSeatHeatThrdLeFnshd_TelmSeatHeatThrdLeFnshd/1 -> PrkgClimaEveMgr_Runnable_sys/173(TelmSeatHeatThrdLeFnshd_TelmSeatHeatThrdLeFnshd_read)
  TelmSeatHeatThrdRiFnshd_TelmSeatHeatThrdRiFnshd/1 -> PrkgClimaEveMgr_Runnable_sys/174(TelmSeatHeatThrdRiFnshd_TelmSeatHeatThrdRiFnshd_read)
  TelmSeatVentnDrvrFnshd_TelmSeatVentnDrvrFnshd/1 -> PrkgClimaEveMgr_Runnable_sys/175(TelmSeatVentnDrvrFnshd_TelmSeatVentnDrvrFnshd_read)
  TelmSeatVentnPassFnshd_TelmSeatVentnPassFnshd/1 -> PrkgClimaEveMgr_Runnable_sys/176(TelmSeatVentnPassFnshd_TelmSeatVentnPassFnshd_read)
  TelmSeatVentnSecLeFnshd_TelmSeatVentnSecLeFnshd/1 -> PrkgClimaEveMgr_Runnable_sys/177(TelmSeatVentnSecLeFnshd_TelmSeatVentnSecLeFnshd_read)
  TelmSeatVentnSecRiFnshd_TelmSeatVentnSecRiFnshd/1 -> PrkgClimaEveMgr_Runnable_sys/178(TelmSeatVentnSecRiFnshd_TelmSeatVentnSecRiFnshd_read)
  TelmSeatVentnThrdLeFnshd_TelmSeatVentnThrdLeFnshd/1 -> PrkgClimaEveMgr_Runnable_sys/179(TelmSeatVentnThrdLeFnshd_TelmSeatVentnThrdLeFnshd_read)
  TelmSeatVentnThrdRiFnshd_TelmSeatVentnThrdRiFnshd/1 -> PrkgClimaEveMgr_Runnable_sys/180(TelmSeatVentnThrdRiFnshd_TelmSeatVentnThrdRiFnshd_read)
  TelmSteerWhlHeatFnshd_TelmSteerWhlHeatFnshd/1 -> PrkgClimaEveMgr_Runnable_sys/181(TelmSteerWhlHeatFnshd_TelmSteerWhlHeatFnshd_read)
  UnLckClimaActvReq_UnLckClimaActvReq/1 -> PrkgClimaEveMgr_Runnable_sys/182(UnLckClimaActvReq_UnLckClimaActvReq_read)
  UnLckHeatActvReq_UnLckHeatActvReq/1 -> PrkgClimaEveMgr_Runnable_sys/183(UnLckHeatActvReq_UnLckHeatActvReq_read)
  VentnForWinOpenGlbActv_VentnForWinOpenGlbActv/1 -> PrkgClimaEveMgr_Runnable_sys/184(VentnForWinOpenGlbActv_VentnForWinOpenGlbActv_read)
  VTMOverTProtectionFnshd_VTMOverTProtectionFnshd/1 -> PrkgClimaEveMgr_Runnable_sys/185(VTMOverTProtectionFnshd_VTMOverTProtectionFnshd_read)
  WarnLvlMsgNgcc_WarnLvlMsgNgcc/1 -> PrkgClimaEveMgr_Runnable_sys/186(WarnLvlMsgNgcc_WarnLvlMsgNgcc_read)
  WarnLvlMsgNgcc_WarnLvlMsgNgcc_IsUpdated/1 -> PrkgClimaEveMgr_Runnable_sys/187(WarnLvlMsgNgcc_WarnLvlMsgNgcc_IsUpdated_read)
  WarnLvlMsgUWB_WarnLvlMsgUWB/1 -> PrkgClimaEveMgr_Runnable_sys/188(WarnLvlMsgUWB_WarnLvlMsgUWB_read)
  WarnLvlMsgUWB_WarnLvlMsgUWB_IsUpdated/1 -> PrkgClimaEveMgr_Runnable_sys/189(WarnLvlMsgUWB_WarnLvlMsgUWB_IsUpdated_read)
  WinOpenLDACReq_WinOpenLDACReq/1 -> PrkgClimaEveMgr_Runnable_sys/190(WinOpenLDACReq_WinOpenLDACReq_read)
  WinOpenLDACReqUWB_WinOpenLDACReqUWB/1 -> PrkgClimaEveMgr_Runnable_sys/191(WinOpenLDACReqUWB_WinOpenLDACReqUWB_read)
  WinPosnStsAtDrvrReNgcc_WinPosnStsAtDrvrReNgcc/1 -> PrkgClimaEveMgr_Runnable_sys/192(WinPosnStsAtDrvrReNgcc_WinPosnStsAtDrvrReNgcc_read)
  WinPosnStsAtPassReNgcc_WinPosnStsAtPassRe/1 -> PrkgClimaEveMgr_Runnable_sys/193(WinPosnStsAtPassReNgcc_WinPosnStsAtPassRe_read)
  WinPosnStsDrv_WinPosnStsAtDrv/1 -> PrkgClimaEveMgr_Runnable_sys/194(WinPosnStsDrv_WinPosnStsAtDrv_read)
  WinPosnStsPass_WinPosnStsAtPass/1 -> PrkgClimaEveMgr_Runnable_sys/195(WinPosnStsPass_WinPosnStsAtPass_read)
  PrkgClimaEveMgr_Runnable_sys/1(HvBattMntnActv_HvBattMntnActv_write) -> HvBattMntnActv_HvBattMntnActv/1
  PrkgClimaEveMgr_Runnable_sys/2(LDACState_LDACState_write) -> LDACState_LDACState/1
  PrkgClimaEveMgr_Runnable_sys/3(MemBookTrvlClimaTi_MemBookTrvlClimaTi_write) -> MemBookTrvlClimaTi_MemBookTrvlClimaTi/1
  PrkgClimaEveMgr_Runnable_sys/4(MemSaveFlgForPrkgClimaEveMgr_MemSaveFlgForPrkgClimaEveMgr_write) -> MemSaveFlgForPrkgClimaEveMgr_MemSaveFlgForPrkgClimaEveMgr/1
  PrkgClimaEveMgr_Runnable_sys/5(MemWrForPrkgClimaEveMgr_MemWrForPrkgClimaEveMgr_write) -> MemWrForPrkgClimaEveMgr_MemWrForPrkgClimaEveMgr/1
  PrkgClimaEveMgr_Runnable_sys/6(PrkgClimaReqExcpSeat_PrkgClimaReqExcpSeat_write) -> PrkgClimaReqExcpSeat_PrkgClimaReqExcpSeat/1
  PrkgClimaEveMgr_Runnable_sys/7(PrkgClimaReqPrio_PrkgClimaReqPrio_write) -> PrkgClimaReqPrio_PrkgClimaReqPrio/1
  PrkgClimaEveMgr_Runnable_sys/8(PrkgOGMAirSrcReq_PrkgOGMAirSrcReq_write) -> PrkgOGMAirSrcReq_PrkgOGMAirSrcReq/1
  PrkgClimaEveMgr_Runnable_sys/9(PrvntWinActvReq_PrvntWinActvReq_write) -> PrvntWinActvReq_PrvntWinActvReq/1
  PrkgClimaEveMgr_Runnable_sys/10(RemClimaEgyExsrcReqPlus_RemClimaEgyExsrcReqPlus_write) -> RemClimaEgyExsrcReqPlus_RemClimaEgyExsrcReqPlus/1
  PrkgClimaEveMgr_Runnable_sys/11(RemOxygenSplyReq_RemOxygenSplyReq_write) -> RemOxygenSplyReq_RemOxygenSplyReq/1
  PrkgClimaEveMgr_Runnable_sys/12(TcamClcltTiFb1_TcamClcltTiFb1_write) -> TcamClcltTiFb1_TcamClcltTiFb1/1
  PrkgClimaEveMgr_Runnable_sys/13(TcamClcltTiFb2_TcamClcltTiFb2_write) -> TcamClcltTiFb2_TcamClcltTiFb2/1
  PrkgClimaEveMgr_Runnable_sys/14(TcamClcltTiFb3_TcamClcltTiFb3_write) -> TcamClcltTiFb3_TcamClcltTiFb3/1
  PrkgClimaEveMgr_Runnable_sys/15(TcamClcltTiFb4_TcamClcltTiFb4_write) -> TcamClcltTiFb4_TcamClcltTiFb4/1
  PrkgClimaEveMgr_Runnable_sys/16(TcamClcltTiFb5_TcamClcltTiFb5_write) -> TcamClcltTiFb5_TcamClcltTiFb5/1
  PrkgClimaEveMgr_Runnable_sys/17(TcamClcltTiFb6_TcamClcltTiFb6_write) -> TcamClcltTiFb6_TcamClcltTiFb6/1
  PrkgClimaEveMgr_Runnable_sys/18(TcamClcltTiFb7_TcamClcltTiFb7_write) -> TcamClcltTiFb7_TcamClcltTiFb7/1
  PrkgClimaEveMgr_Runnable_sys/19(TcsOvrheatdProtnReq_TcsOvrheatdProtnReq_write) -> TcsOvrheatdProtnReq_TcsOvrheatdProtnReq/1
  PrkgClimaEveMgr_Runnable_sys/20(TelmBookClimaReqHmiCmptmtTSpSpclStrtReq_TelmBookClimaReqHmiCmptmtTSpSpclStrtReq_write) -> TelmBookClimaReqHmiCmptmtTSpSpclStrtReq_TelmBookClimaReqHmiCmptmtTSpSpclStrtReq/1
  PrkgClimaEveMgr_Runnable_sys/21(TelmBookClimaReqOnOffNoReqStrtReq_TelmBookClimaReqOnOffNoReqStrtReq_write) -> TelmBookClimaReqOnOffNoReqStrtReq_TelmBookClimaReqOnOffNoReqStrtReq/1
  PrkgClimaEveMgr_Runnable_sys/22(TelmBookClimaReqTempRangeStrtReq_TelmBookClimaReqTempRangeStrtReq_write) -> TelmBookClimaReqTempRangeStrtReq_TelmBookClimaReqTempRangeStrtReq/1
  PrkgClimaEveMgr_Runnable_sys/23(TelmBookClimaTmrStrtReq_TelmBookClimaTmrStrtReq_write) -> TelmBookClimaTmrStrtReq_TelmBookClimaTmrStrtReq/1
  PrkgClimaEveMgr_Runnable_sys/24(TelmBookSeatHeatClimalReqDrvHeatClimaLvlStrtReq_TelmBookSeatHeatClimalReqDrvHeatClimaLvlStrtReq_write) -> TelmBookSeatHeatClimalReqDrvHeatClimaLvlStrtReq_TelmBookSeatHeatClimalReqDrvHeatClimaLvlStrtReq/1
  PrkgClimaEveMgr_Runnable_sys/25(TelmBookSeatHeatClimalReqPassHeatClimaLvlStrtReq_TelmBookSeatHeatClimalReqPassHeatClimaLvlStrtReq_write) -> TelmBookSeatHeatClimalReqPassHeatClimaLvlStrtReq_TelmBookSeatHeatClimalReqPassHeatClimaLvlStrtReq/1
  PrkgClimaEveMgr_Runnable_sys/26(TelmBookSeatHeatClimalReqSecLeHeatClimaLvlStrtReq_TelmBookSeatHeatClimalReqSecLeHeatClimaLvlStrtReq_write) -> TelmBookSeatHeatClimalReqSecLeHeatClimaLvlStrtReq_TelmBookSeatHeatClimalReqSecLeHeatClimaLvlStrtReq/1
  PrkgClimaEveMgr_Runnable_sys/27(TelmBookSeatHeatClimalReqSecRiHeatClimaLvlStrtReq_TelmBookSeatHeatClimalReqSecRiHeatClimaLvlStrtReq_write) -> TelmBookSeatHeatClimalReqSecRiHeatClimaLvlStrtReq_TelmBookSeatHeatClimalReqSecRiHeatClimaLvlStrtReq/1
  PrkgClimaEveMgr_Runnable_sys/28(TelmBookSeatHeatClimalReqThrdLeHeatClimaLvlStrtReq_TelmBookSeatHeatClimalReqThrdLeHeatClimaLvlStrtReq_write) -> TelmBookSeatHeatClimalReqThrdLeHeatClimaLvlStrtReq_TelmBookSeatHeatClimalReqThrdLeHeatClimaLvlStrtReq/1
  PrkgClimaEveMgr_Runnable_sys/29(TelmBookSeatHeatClimalReqThrdRiHeatClimaLvlStrtReq_TelmBookSeatHeatClimalReqThrdRiHeatClimaLvlStrtReq_write) -> TelmBookSeatHeatClimalReqThrdRiHeatClimaLvlStrtReq_TelmBookSeatHeatClimalReqThrdRiHeatClimaLvlStrtReq/1
  PrkgClimaEveMgr_Runnable_sys/30(TelmBookSeatVentnClimalReqDrvVentnClimaLvlStrtReq_TelmBookSeatVentnClimalReqDrvVentnClimaLvlStrtReq_write) -> TelmBookSeatVentnClimalReqDrvVentnClimaLvlStrtReq_TelmBookSeatVentnClimalReqDrvVentnClimaLvlStrtReq/1
  PrkgClimaEveMgr_Runnable_sys/31(TelmBookSeatVentnClimalReqPassVentnClimaLvlStrtReq_TelmBookSeatVentnClimalReqPassVentnClimaLvlStrtReq_write) -> TelmBookSeatVentnClimalReqPassVentnClimaLvlStrtReq_TelmBookSeatVentnClimalReqPassVentnClimaLvlStrtReq/1
  PrkgClimaEveMgr_Runnable_sys/32(TelmBookSeatVentnClimalReqSecLeVentnClimaLvlStrtReq_TelmBookSeatVentnClimalReqSecLeVentnClimaLvlStrtReq_write) -> TelmBookSeatVentnClimalReqSecLeVentnClimaLvlStrtReq_TelmBookSeatVentnClimalReqSecLeVentnClimaLvlStrtReq/1
  PrkgClimaEveMgr_Runnable_sys/33(TelmBookSeatVentnClimalReqSecRiVentnClimaLvlStrtReq_TelmBookSeatVentnClimalReqSecRiVentnClimaLvlStrtReq_write) -> TelmBookSeatVentnClimalReqSecRiVentnClimaLvlStrtReq_TelmBookSeatVentnClimalReqSecRiVentnClimaLvlStrtReq/1
  PrkgClimaEveMgr_Runnable_sys/34(TelmBookSeatVentnClimalReqThrdLeVentnClimaLvlStrtReq_TelmBookSeatVentnClimalReqThrdLeVentnClimaLvlStrtReq_write) -> TelmBookSeatVentnClimalReqThrdLeVentnClimaLvlStrtReq_TelmBookSeatVentnClimalReqThrdLeVentnClimaLvlStrtReq/1
  PrkgClimaEveMgr_Runnable_sys/35(TelmBookSeatVentnClimalReqThrdRiVentnClimaLvlStrtReq_TelmBookSeatVentnClimalReqThrdRiVentnClimaLvlStrtReq_write) -> TelmBookSeatVentnClimalReqThrdRiVentnClimaLvlStrtReq_TelmBookSeatVentnClimalReqThrdRiVentnClimaLvlStrtReq/1
  PrkgClimaEveMgr_Runnable_sys/36(TelmBookSteerWhlHeatClimalReqStrtReq_TelmBookSteerWhlHeatClimalReqStrtReq_write) -> TelmBookSteerWhlHeatClimalReqStrtReq_TelmBookSteerWhlHeatClimalReqStrtReq/1
  PrkgClimaEveMgr_Runnable_sys/37(TelmSeatVentnThrdReq_TelmSeatVentnThrdReq_write) -> TelmSeatVentnThrdReq_TelmSeatVentnThrdReq/1
  PrkgClimaEveMgr_Runnable_sys/38(UnLckClimaActvReqSt_UnLckClimaActvReqSt_write) -> UnLckClimaActvReqSt_UnLckClimaActvReqSt/1
  PrkgClimaEveMgr_Runnable_sys/39(UnLckHeatActvReqSt_UnLckHeatActvReqSt_write) -> UnLckHeatActvReqSt_UnLckHeatActvReqSt/1
  PrkgClimaEveMgr_Runnable_sys/40(ActvRqrdForPostPrkgClima_ActvRqrdForPostPrkgClima_write) -> ActvRqrdForPostPrkgClima_ActvRqrdForPostPrkgClima/1
  PrkgClimaEveMgr_Runnable_sys/41(ClimaOvrHeatProWarnIni_ClimaOvrHeatProWarnIni_write) -> ClimaOvrHeatProWarnIni_ClimaOvrHeatProWarnIni/1
  PrkgClimaEveMgr_Runnable_sys/42(ClimaOvrHeatStrtReq_ClimaOvrHeatStrtReq_write) -> ClimaOvrHeatStrtReq_ClimaOvrHeatStrtReq/1
  PrkgClimaEveMgr_Runnable_sys/43(ClimaStrtTi_ClimaStrtTi_write) -> ClimaStrtTi_ClimaStrtTi/1
  PrkgClimaEveMgr_Runnable_sys/44(CSDUsgChgeOffReq_CSDUsgChgeOffReq_write) -> CSDUsgChgeOffReq_CSDUsgChgeOffReq/1
  PrkgClimaEveMgr_Runnable_sys/45(CSDUsgChgeOnReq_CSDUsgChgeOnReq_write) -> CSDUsgChgeOnReq_CSDUsgChgeOnReq/1
  PrkgClimaEveMgr_Runnable_sys/46(EcuDogKeepActv_EcuDogKeepActv_write) -> EcuDogKeepActv_EcuDogKeepActv/1
  PrkgClimaEveMgr_Runnable_sys/47(EcuDogSlpReq_EcuDogSlpReq_write) -> EcuDogSlpReq_EcuDogSlpReq/1
  PrkgClimaEveMgr_Runnable_sys/48(EcuDogSlpSetTi_EcuDogSlpSetTi_write) -> EcuDogSlpSetTi_EcuDogSlpSetTi/1
  PrkgClimaEveMgr_Runnable_sys/49(ErsCmdByKeyStrtReq_ErsCmdByKeyStrtReq_write) -> ErsCmdByKeyStrtReq_ErsCmdByKeyStrtReq/1
  PrkgClimaEveMgr_Runnable_sys/50(ErsRunTimelStrtReq_ErsRunTimelStrtReq_write) -> ErsRunTimelStrtReq_ErsRunTimelStrtReq/1
  PrkgClimaEveMgr_Runnable_sys/51(HeatrTypForPrkgClima_HeatrTypForPrkgClima_write) -> HeatrTypForPrkgClima_HeatrTypForPrkgClima/1
  PrkgClimaEveMgr_Runnable_sys/52(HMIExtVentFanVolReqStrtReq_HMIExtVentFanVolReqStrtReq_write) -> HMIExtVentFanVolReqStrtReq_HMIExtVentFanVolReqStrtReq/1
  PrkgClimaEveMgr_Runnable_sys/53(HMIExtVentonoffReqStrtReq_HMIExtVentonoffReqStrtReq_write) -> HMIExtVentonoffReqStrtReq_HMIExtVentonoffReqStrtReq/1
  PrkgClimaEveMgr_Runnable_sys/54(HMIExtVentTSpReqStrtReq_HMIExtVentTSpReqStrtReq_write) -> HMIExtVentTSpReqStrtReq_HMIExtVentTSpReqStrtReq/1
  PrkgClimaEveMgr_Runnable_sys/55(HMIExtVentTStsReqStrtReq_HMIExtVentTStsReqStrtReq_write) -> HMIExtVentTStsReqStrtReq_HMIExtVentTStsReqStrtReq/1
  PrkgClimaEveMgr_Runnable_sys/56(HvBattForPrkgClima_HvBattForPrkgClima_write) -> HvBattForPrkgClima_HvBattForPrkgClima/1
  PrkgClimaEveMgr_Runnable_sys/57(IntCo2MontrStrtReq_IntCo2MontrStrtReq_write) -> IntCo2MontrStrtReq_IntCo2MontrStrtReq/1
  PrkgClimaEveMgr_Runnable_sys/58(NetReqFromPrkgClimaEveMgr_NetReqFromPrkgClimaEveMgr_write) -> NetReqFromPrkgClimaEveMgr_NetReqFromPrkgClimaEveMgr/1
  PrkgClimaEveMgr_Runnable_sys/59(ObsvrActvnReqFromPrkgClima_ObsvrActvnReqFromPrkgClima_write) -> ObsvrActvnReqFromPrkgClima_ObsvrActvnReqFromPrkgClima/1
  PrkgClimaEveMgr_Runnable_sys/60(OffRqrdForPrkgClima_OffRqrdForPrkgClima_write) -> OffRqrdForPrkgClima_OffRqrdForPrkgClima/1
  PrkgClimaEveMgr_Runnable_sys/61(OffRqrdForRemClima_OffRqrdForRemClima_write) -> OffRqrdForRemClima_OffRqrdForRemClima/1
  PrkgClimaEveMgr_Runnable_sys/62(PostDrvgClimaAvl_Ngcc_PostDrvgClimaAvl_write) -> PostDrvgClimaAvl_Ngcc_PostDrvgClimaAvl/1
  PrkgClimaEveMgr_Runnable_sys/63(PrePrkgClimaNotAvlForHvSysWoU_PrePrkgClimaNotAvlForHvSysWoU_write) -> PrePrkgClimaNotAvlForHvSysWoU_PrePrkgClimaNotAvlForHvSysWoU/1
  PrkgClimaEveMgr_Runnable_sys/64(PrkgCDSAvl_PrkgCDSAvl_write) -> PrkgCDSAvl_PrkgCDSAvl/1
  PrkgClimaEveMgr_Runnable_sys/65(PrkgClimaAllwdForBattULo_PrkgClimaAllwdForBattULo_write) -> PrkgClimaAllwdForBattULo_PrkgClimaAllwdForBattULo/1
  PrkgClimaEveMgr_Runnable_sys/66(PrkgClimaAllwdForSwtHeatrTyp_PrkgClimaAllwdForSwtHeatrTyp_write) -> PrkgClimaAllwdForSwtHeatrTyp_PrkgClimaAllwdForSwtHeatrTyp/1
  PrkgClimaEveMgr_Runnable_sys/67(RemClimaallExsrcReq_RemClimaallExsrcReq_write) -> RemClimaallExsrcReq_RemClimaallExsrcReq/1
  PrkgClimaEveMgr_Runnable_sys/68(RemClimaEgyExsrcReq_RemClimaEgyExsrcReq_write) -> RemClimaEgyExsrcReq_RemClimaEgyExsrcReq/1
  PrkgClimaEveMgr_Runnable_sys/69(RemClimaTmrStrtReq_RemClimaTmrStrtReq_write) -> RemClimaTmrStrtReq_RemClimaTmrStrtReq/1
  PrkgClimaEveMgr_Runnable_sys/70(RemStrtExtnTiStrtReq_RemStrtExtnTiStrtReq_write) -> RemStrtExtnTiStrtReq_RemStrtExtnTiStrtReq/1
  PrkgClimaEveMgr_Runnable_sys/71(RemStrtHvCtrlStrtReq_RemStrtHvCtrlStrtReq_write) -> RemStrtHvCtrlStrtReq_RemStrtHvCtrlStrtReq/1
  PrkgClimaEveMgr_Runnable_sys/72(RemVentReqStrtReq_RemVentReqStrtReq_write) -> RemVentReqStrtReq_RemVentReqStrtReq/1
  PrkgClimaEveMgr_Runnable_sys/73(ReqForSwtHeatrTypFromEveMgr_ReqForSwtHeatrTypFromEveMgr_write) -> ReqForSwtHeatrTypFromEveMgr_ReqForSwtHeatrTypFromEveMgr/1
  PrkgClimaEveMgr_Runnable_sys/74(RtBasSdlForTmrPrePrkgClima_RtBasSdlForTmrPrePrkgClima_write) -> RtBasSdlForTmrPrePrkgClima_RtBasSdlForTmrPrePrkgClima/1
  PrkgClimaEveMgr_Runnable_sys/75(RtCmftForImdtPrePrkgClima_RtCmftForImdtPrePrkgClima_write) -> RtCmftForImdtPrePrkgClima_RtCmftForImdtPrePrkgClima/1
  PrkgClimaEveMgr_Runnable_sys/76(RtCmftForPostPrkgClima_RtCmftForPostPrkgClima_write) -> RtCmftForPostPrkgClima_RtCmftForPostPrkgClima/1
  PrkgClimaEveMgr_Runnable_sys/77(RtCmftForPreClng_RtCmftForPreClng_write) -> RtCmftForPreClng_RtCmftForPreClng/1
  PrkgClimaEveMgr_Runnable_sys/78(RtCmftForTmrPrePrkgClima_RtCmftForTmrPrePrkgClima_write) -> RtCmftForTmrPrePrkgClima_RtCmftForTmrPrePrkgClima/1
  PrkgClimaEveMgr_Runnable_sys/79(RtMaxForPrePrkgClima_RtMaxForPrePrkgClima_write) -> RtMaxForPrePrkgClima_RtMaxForPrePrkgClima/1
  PrkgClimaEveMgr_Runnable_sys/80(SeatHeatForPrkgClimaEnad_SeatHeatForPrkgClimaEnad_write) -> SeatHeatForPrkgClimaEnad_SeatHeatForPrkgClimaEnad/1
  PrkgClimaEveMgr_Runnable_sys/81(SteerWhlHeatgForPrkgClimaEnad_SteerWhlHeatgForPrkgClimaEnad_write) -> SteerWhlHeatgForPrkgClimaEnad_SteerWhlHeatgForPrkgClimaEnad/1
  PrkgClimaEveMgr_Runnable_sys/82(StrtRqrdForDirForPrePrkgClima_StrtRqrdForDirForPrePrkgClima_write) -> StrtRqrdForDirForPrePrkgClima_StrtRqrdForDirForPrePrkgClima/1
  PrkgClimaEveMgr_Runnable_sys/83(StrtRqrdForPreClng_StrtRqrdForPreClng_write) -> StrtRqrdForPreClng_StrtRqrdForPreClng/1
  PrkgClimaEveMgr_Runnable_sys/84(StrtRqrdForTmrForPrePrkgClima_StrtRqrdForTmrForPrePrkgClima_write) -> StrtRqrdForTmrForPrePrkgClima_StrtRqrdForTmrForPrePrkgClima/1
  PrkgClimaEveMgr_Runnable_sys/85(SuspStrtAllwdForPrkgClima_SuspStrtAllwdForPrkgClima_write) -> SuspStrtAllwdForPrkgClima_SuspStrtAllwdForPrkgClima/1
  PrkgClimaEveMgr_Runnable_sys/86(TelmClimaExtVentnLvlStrtReq_TelmClimaExtVentnLvlStrtReq_write) -> TelmClimaExtVentnLvlStrtReq_TelmClimaExtVentnLvlStrtReq/1
  PrkgClimaEveMgr_Runnable_sys/87(TelmClimaExtVentnReqStrtReq_TelmClimaExtVentnReqStrtReq_write) -> TelmClimaExtVentnReqStrtReq_TelmClimaExtVentnReqStrtReq/1
  PrkgClimaEveMgr_Runnable_sys/88(TelmClimaExtVentnTempRangeStrtReq_TelmClimaExtVentnTempRangeStrtReq_write) -> TelmClimaExtVentnTempRangeStrtReq_TelmClimaExtVentnTempRangeStrtReq/1
  PrkgClimaEveMgr_Runnable_sys/89(TelmClimaExtVentnTSpSpclStrtReq_TelmClimaExtVentnTSpSpclStrtReq_write) -> TelmClimaExtVentnTSpSpclStrtReq_TelmClimaExtVentnTSpSpclStrtReq/1
  PrkgClimaEveMgr_Runnable_sys/90(UpdRtTotForTmrForPrePrkgClima_UpdRtTotForTmrForPrePrkgClima_write) -> UpdRtTotForTmrForPrePrkgClima_UpdRtTotForTmrForPrePrkgClima/1
  PrkgClimaEveMgr_Runnable_sys/91(WarnForHybWoU_WarnForHybWoU_write) -> WarnForHybWoU_WarnForHybWoU/1
  PrkgClimaEveMgr_Runnable_sys/92(WarnForHybWoUForTmr_WarnForHybWoUForTmr_write) -> WarnForHybWoUForTmr_WarnForHybWoUForTmr/1
  PrkgClimaEveMgr_Runnable_sys/93(WarnForPreCdngFctNotAvl_WarnForPreCdngFctNotAvl_write) -> WarnForPreCdngFctNotAvl_WarnForPreCdngFctNotAvl/1
  PrkgClimaEveMgr_Runnable_sys/94(WarnForTmrPreCdngFctNotAvl_WarnForTmrPreCdngFctNotAvl_write) -> WarnForTmrPreCdngFctNotAvl_WarnForTmrPreCdngFctNotAvl/1
  PrkgClimaEveMgr_Runnable_sys/95(IntCo2SetStartTime_IntCo2SetStartTime_write) -> IntCo2SetStartTime_IntCo2SetStartTime/1
  PrkgClimaEveMgr_Runnable_sys/96(IntCo2SetStopTime_IntCo2SetStopTime_write) -> IntCo2SetStopTime_IntCo2SetStopTime/1
  PrkgClimaEveMgr_Runnable_sys/97(RemClimaTempStrtReq_RemClimaTempStrtReq_write) -> RemClimaTempStrtReq_RemClimaTempStrtReq/1
  PrkgClimaEveMgr_Runnable/1 -> PrkgClimaEveMgr_Runnable_sys/Trigger
